simulink model "CDF_VSVPWM_Grid_Parallel"  (R2024b) (continued)
== system CDF_VSVPWM_Grid_Parallel/VSVPWM == (continued)
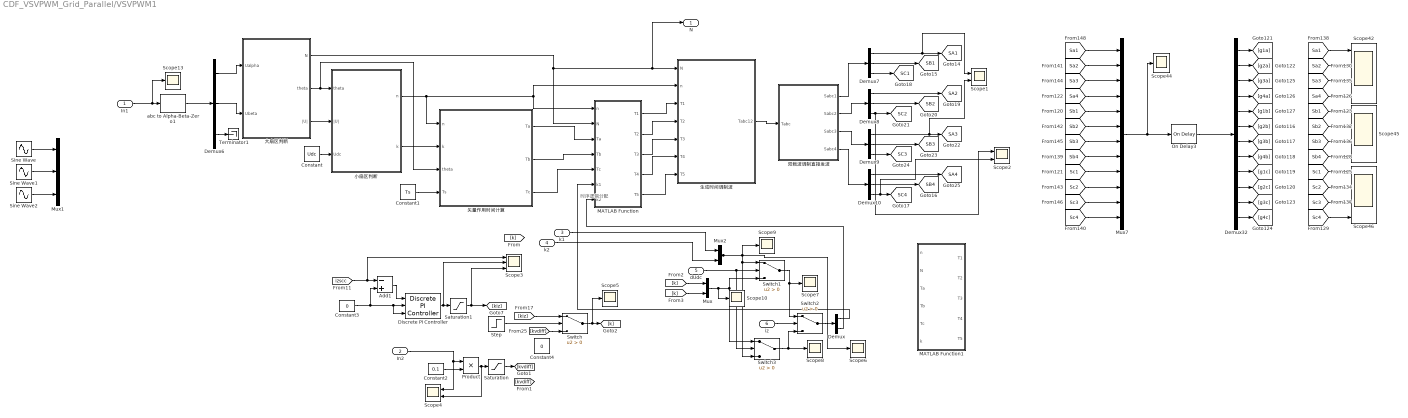
== system CDF_VSVPWM_Grid_Parallel/VSVPWM1 ==
  note: 时序逻辑分配
  In1 : Inport
  In2 : Inport  Port=2
  k1 : Inport  Port=3
  k2 : Inport  Port=4
  dUdc : Inport  Port=5
  iz : Inport  Port=6
  Add1 : Sum  Inputs=-+
  Constant : Constant  Value=Udc
  Constant1 : Constant  Value=Ts
  Constant2 : Constant  Value=0.1
  Constant3 : Constant  Value=0
  Constant4 : Constant  Value=0
  Demux : Demux  Outputs=2
  Demux{6..10} (5 alike) : Demux  Outputs=3
  Demux32 : Demux  Outputs=12
  Discrete PI Controller : eeDiscretePi/Discrete PI Controller  Kp=5  Ki=0  Kaw=1  x0=0  Ts=-1
  MATLAB Function : MATLAB Function  TreatAsAtomicUnit=on
  MATLAB Function1 : MATLAB Function  TreatAsAtomicUnit=on
  Mux : Mux  Inputs=2
  Mux1 : Mux  Inputs=3
  Mux2 : Mux  Inputs=2
  Mux7 : Mux  Inputs=12
  On Delay3 : spsOnDelayLib/On Delay  DelayType=On delay  delay=250e-9  ic=0  Ts=0
  Product : Product
  Saturation : Saturate  UpperLimit=1  LowerLimit=-1
  Saturation1 : Saturate  UpperLimit=1  LowerLimit=-1
  Scope1 : Scope  NumInputPorts=2
  Scope{5..10,13,44} (8 alike) : Scope
  Scope2 : Scope  NumInputPorts=2
  Scope3 : Scope  NumInputPorts=3
  Scope4 : Scope  NumInputPorts=2
  Scope42 : Scope  NumInputPorts=4
  Scope45 : Scope  NumInputPorts=4
  Scope46 : Scope  NumInputPorts=4
  Sine Wave : Sin  Amplitude=m*Udc/sqrt(3)  Frequency=100*pi  SampleTime=0
  Sine Wave1 : Sin  Amplitude=m*Udc/sqrt(3)  Frequency=100*pi  Phase=-2*pi/3  SampleTime=0
  Sine Wave2 : Sin  Amplitude=m*Udc/sqrt(3)  Frequency=100*pi  Phase=2*pi/3  SampleTime=0
  Step : Step  SampleTime=0
  Switch{,1..3} (4 alike) : Switch  Criteria=u2 > Threshold  SaturateOnIntegerOverflow=off  InputSameDT=off
  Terminator1 : Terminator
  abc to Alpha-Beta-Zero1 : spsabctoAlphaBetaZeroLib/abc to Alpha-Beta-Zero
  双载波调制直接发波 : Subsystem
  大扇区判断 : Subsystem
  小扇区判断 : Subsystem
  生成时间调制波 : Subsystem
  矢量作用时间计算 : Subsystem
  N : Outport
  tag scope: SA1=global, SA2=global, SA3=global, SA4=global, SB1=global, SB2=global, SB3=global, SB4=global, SC1=global, SC2=global, SC3=global, SC4=global
  wires:
  大扇区判断/2(theta) -> 矢量作用时间计算/3(theta), 小扇区判断/1(theta)
  大扇区判断/3(|U|) -> 小扇区判断/2(|U|)
  Constant/1 -> 小扇区判断/3(Udc)
  Demux6/2 -> 大扇区判断/2(Ubeta)
  abc to Alpha-Beta-Zero1/1 -> Demux6/1
  In1/1 -> Scope13/1, abc to Alpha-Beta-Zero1/1
  Demux6/1 -> 大扇区判断/1(Ualpha)
  小扇区判断/2(k) -> 矢量作用时间计算/2(k)
  小扇区判断/1(n) -> 矢量作用时间计算/1(n), MATLAB Function/1(n), 生成时间调制波/2(n)
  Constant1/1 -> 矢量作用时间计算/4(Ts)
  大扇区判断/1(N) -> MATLAB Function/2(N), N/1, 生成时间调制波/1(N)
  生成时间调制波/1(Tabc12) -> 双载波调制直接发波/1(Tabc)
  Demux9/3 -> [SC3]
  Demux9/2 -> [SB3]
  Demux8/1 -> [SA2]
  Demux10/2 -> [SB4]
  Demux10/3 -> Scope2/2, [SC4]
  Demux7/1 -> Scope1/1, [SA1]
  Demux10/1 -> [SA4]
  Demux8/2 -> [SB2]
  Demux7/2 -> [SB1]
  Demux7/3 -> [SC1]
  Demux9/1 -> Scope1/2, [SA3]
  Demux8/3 -> Scope2/1, [SC2]
  双载波调制直接发波/1(Sabc1) -> Demux7/1
  双载波调制直接发波/2(Sabc2) -> Demux8/1
  双载波调制直接发波/3(Sabc3) -> Demux9/1
  双载波调制直接发波/4(Sabc4) -> Demux10/1
  Demux6/3 -> Terminator1/1
  [Sc3] -> Scope46/3
  [Sa3] -> Scope42/3
  Demux32/11 -> [g3c]
  Demux32/2 -> [g2a]
  On Delay3/1 -> Demux32/1
  Demux32/5 -> [g1b]
  Demux32/12 -> [g4c]
  Demux32/1 -> [g1a]
  Demux32/10 -> [g2c]
  Demux32/7 -> [g3b]
  Demux32/8 -> [g4b]
  Demux32/6 -> [g2b]
  [Sb3] -> Mux7/7
  Mux7/1 -> On Delay3/1, Scope44/1
  [Sc1] -> Mux7/9
  [Sb1] -> Scope45/1
  [Sb2] -> Scope45/2
  [Sa2] -> Scope42/2
  [Sb3] -> Scope45/3
  Demux32/9 -> [g1c]
  [Sb4] -> Scope45/4
  Demux32/3 -> [g3a]
  [Sb1] -> Mux7/5
  [Sa1] -> Mux7/1
  [Sa3] -> Mux7/3
  [Sb2] -> Mux7/6
  [Sa2] -> Mux7/2
  [Sb4] -> Mux7/8
  Demux32/4 -> [g4a]
  [Sa4] -> Mux7/4
  [Sc4] -> Mux7/12
  [Sc4] -> Scope46/4
  [Sc2] -> Scope46/2
  [Sa1] -> Scope42/1
  [Sc3] -> Mux7/11
  [Sc2] -> Mux7/10
  [Sa4] -> Scope42/4
  [Sc1] -> Scope46/1
  Sine Wave1/1 -> Mux1/2
  Sine Wave2/1 -> Mux1/3
  Sine Wave/1 -> Mux1/1
  Saturation/1 -> [kvdiff]
  Add1/1 -> Discrete PI Controller/1
  Switch/1 -> Scope5/1, [k]
  Constant2/1 -> Product/2
  In2/1 -> Scope4/1, Product/1
  Step/1 -> Switch/2
  Discrete PI Controller/1 -> Scope3/2, Saturation1/1
  Saturation1/1 -> Scope3/3, [kiz]
  Product/1 -> Scope4/2, Saturation/1
  Constant3/1 -> Add1/2, Discrete PI Controller/3, Discrete PI Controller/2
  [izscc] -> Scope3/1, Add1/1
  [kiz] -> Switch/1
  [kvdiff] -> Switch/3
  矢量作用时间计算/1(Ta) -> MATLAB Function/3(Ta)
  矢量作用时间计算/2(Tb) -> MATLAB Function/4(Tb)
  矢量作用时间计算/3(Tc) -> MATLAB Function/5(Tc)
  MATLAB Function/1(T1) -> 生成时间调制波/3(T1)
  MATLAB Function/2(T2) -> 生成时间调制波/4(T2)
  MATLAB Function/3(T3) -> 生成时间调制波/5(T3)
  MATLAB Function/4(T4) -> 生成时间调制波/6(T4)
  MATLAB Function/5(T5) -> 生成时间调制波/7(T5)
  iz/1 -> Switch2/2
  [k] -> Mux/1
  Switch1/1 -> Switch2/1, Scope7/1
  Mux/1 -> Scope10/1, Switch3/1, Switch1/3
  Mux2/1 -> Switch3/3, Switch1/1, Scope9/1, Demux/1
  Switch3/1 -> Scope8/1, Switch2/3
  [k] -> Mux/2
  Switch2/1 -> Scope6/1
  dUdc/1 -> Switch1/2, Switch3/2
  Demux/1 -> MATLAB Function/6(k1)
  Demux/2 -> MATLAB Function/7(k2)
  k1/1 -> Mux2/1
  k2/1 -> Mux2/2

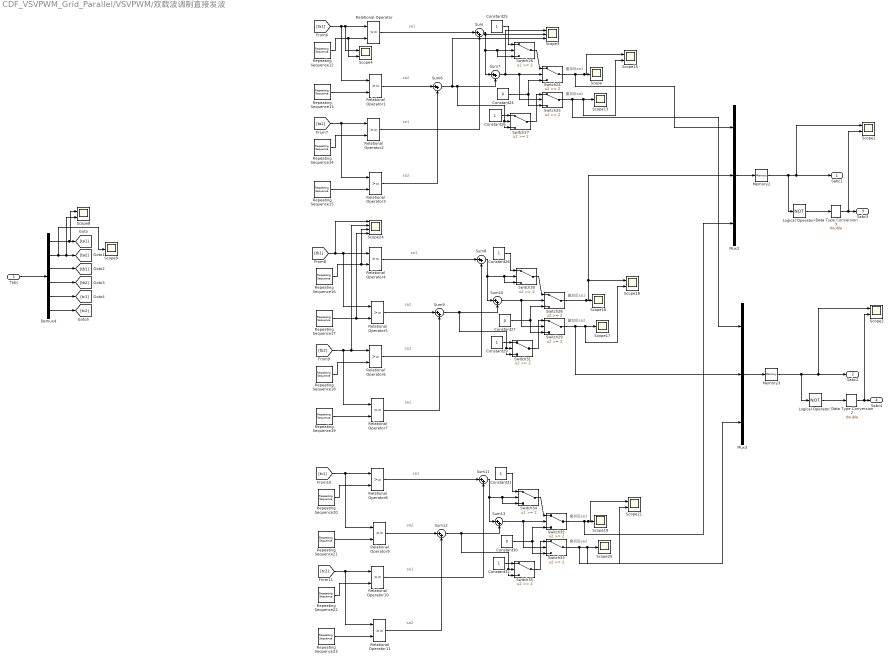
== system CDF_VSVPWM_Grid_Parallel/VSVPWM/双载波调制直接发波 ==
  note: 叠加后sa1
  note: sa1
  note: sa2
  note: sa1
  note: sa2
  note: 叠加后sa2
  note: 叠加后sa1
  note: 叠加后sa2
  note: sa1
  note: sa1
  note: sa2
  note: sa2
  note: 叠加后sa1
  note: 叠加后sa2
  note: sa1
  note: sa1
  note: sa2
  note: sa2
  Tabc : Inport
  Constant24 : Constant  Value=0
  Constant{25..26,28..29,31..32} (6 alike) : Constant
  Constant27 : Constant  Value=0
  Constant30 : Constant  Value=0
  Data Type Conversion2 : DataTypeConversion  OutDataTypeStr=double  SaturateOnIntegerOverflow=off  RndMeth=Floor
  Data Type Conversion3 : DataTypeConversion  OutDataTypeStr=double  SaturateOnIntegerOverflow=off  RndMeth=Floor
  Demux4 : Demux  Outputs=6
  Logical Operator1 : Logic  Operator=NOT  AllPortsSameDT=off  OutDataTypeStr=boolean
  Logical Operator4 : Logic  Operator=NOT  AllPortsSameDT=off  OutDataTypeStr=boolean
  Memory2 : Memory
  Memory3 : Memory
  Mux2 : Mux  Inputs=3
  Mux3 : Mux  Inputs=3
  Relational Operator{,1..11} (12 alike) : RelationalOperator  OutDataTypeStr=boolean  RndMeth=Simplest  InputSameDT=off
  Repeating Sequence{12,14,16,18,20,22} (6 alike) : simulink/Sources/Repeating Sequence  rep_seq_t=[0 Ts/2 Ts]  rep_seq_y=[0 1 0]
  Repeating Sequence{13,15,17,19,21,23} (6 alike) : simulink/Sources/Repeating Sequence  rep_seq_t=[0 Ts/2 Ts]  rep_seq_y=[-1 0 -1]
  Scope{,9,13,16..17,19..20} (7 alike) : Scope
  Scope{1..2,4,8,15,18,21} (7 alike) : Scope  NumInputPorts=2
  Scope24 : Scope  NumInputPorts=4
  Scope3 : Scope  NumInputPorts=3
  Sum{,6..13} (9 alike) : Sum  Inputs=|++
  Switch{24..35} (12 alike) : Switch  Threshold=2  SaturateOnIntegerOverflow=off  InputSameDT=off
  Sabc1 : Outport
  Sabc2 : Outport  Port=2
  Sabc3 : Outport  Port=3
  Sabc4 : Outport  Port=4
  wires:
  Tabc/1 -> Demux4/1
  Demux4/1 -> Scope8/1, [ta1]
  Demux4/2 -> Scope9/1, Scope8/2, [ta2]
  Demux4/3 -> [tb1]
  Demux4/4 -> [tb2]
  Demux4/5 -> [tc1]
  Demux4/6 -> [tc2]
  [ta1] -> Relational Operator1/1, Scope4/1, Relational Operator/1
  Repeating Sequence12/1 -> Scope4/2, Relational Operator/2
  Repeating Sequence13/1 -> Relational Operator1/2
  Relational Operator/1 -> Sum/1
  Relational Operator1/1 -> Sum6/1
  Relational Operator2/1 -> Sum/2
  [ta2] -> Relational Operator3/1, Relational Operator2/1
  Repeating Sequence15/1 -> Relational Operator3/2
  Repeating Sequence14/1 -> Relational Operator2/2
  Relational Operator3/1 -> Sum6/2
  Sum/1 -> Switch26/3, Switch26/2, Sum7/1, Scope3/2
  Sum6/1 -> Scope3/3, Switch27/3, Switch27/2, Sum7/2
  Sum7/1 -> Scope3/1, Switch25/2, Switch24/2
  Constant24/1 -> Switch25/3, Switch24/3
  Switch24/1 -> Mux2/1, Scope15/1, Scope/1
  Switch25/1 -> Mux3/1, Scope15/2, Scope13/1
  Constant25/1 -> Switch26/1
  Switch26/1 -> Switch24/1
  Switch27/1 -> Switch25/1
  Constant26/1 -> Switch27/1
  Switch28/1 -> Mux2/2, Scope18/1, Scope16/1
  Switch30/1 -> Switch28/1
  Constant28/1 -> Switch30/1
  Relational Operator7/1 -> Sum9/2
  Sum8/1 -> Switch30/3, Switch30/2, Sum10/1
  Relational Operator4/1 -> Sum8/1
  Repeating Sequence17/1 -> Relational Operator5/2, Scope24/4
  Sum10/1 -> Switch29/2, Switch28/2
  [tb1] -> Scope24/1, Relational Operator5/1, Relational Operator4/1
  Relational Operator5/1 -> Sum9/1
  Switch29/1 -> Mux3/2, Scope18/2, Scope17/1
  Repeating Sequence16/1 -> Scope24/3, Relational Operator4/2
  Sum9/1 -> Switch31/3, Switch31/2, Sum10/2
  Relational Operator6/1 -> Sum8/2
  Repeating Sequence18/1 -> Relational Operator6/2
  Constant27/1 -> Switch29/3, Switch28/3
  Repeating Sequence19/1 -> Relational Operator7/2
  [tb2] -> Scope24/2, Relational Operator6/1, Relational Operator7/1
  Switch31/1 -> Switch29/1
  Constant29/1 -> Switch31/1
  Switch32/1 -> Mux2/3, Scope21/1, Scope19/1
  Switch34/1 -> Switch32/1
  Constant31/1 -> Switch34/1
  Relational Operator11/1 -> Sum12/2
  Sum11/1 -> Switch34/3, Switch34/2, Sum13/1
  Relational Operator8/1 -> Sum11/1
  Repeating Sequence21/1 -> Relational Operator9/2
  Sum13/1 -> Switch33/2, Switch32/2
  [tc1] -> Relational Operator9/1, Relational Operator8/1
  Relational Operator9/1 -> Sum12/1
  Switch33/1 -> Mux3/3, Scope21/2, Scope20/1
  Repeating Sequence20/1 -> Relational Operator8/2
  Sum12/1 -> Switch35/3, Switch35/2, Sum13/2
  Relational Operator10/1 -> Sum11/2
  Repeating Sequence22/1 -> Relational Operator10/2
  Constant30/1 -> Switch33/3, Switch32/3
  Repeating Sequence23/1 -> Relational Operator11/2
  [tc2] -> Relational Operator11/1, Relational Operator10/1
  Switch35/1 -> Switch33/1
  Constant32/1 -> Switch35/1
  Logical Operator1/1 -> Data Type Conversion2/1
  Logical Operator4/1 -> Data Type Conversion3/1
  Mux2/1 -> Memory2/1
  Data Type Conversion3/1 -> Scope1/2, Sabc3/1
  Mux3/1 -> Memory3/1
  Data Type Conversion2/1 -> Scope2/2, Sabc4/1
  Memory2/1 -> Logical Operator4/1, Scope1/1, Sabc1/1
  Memory3/1 -> Logical Operator1/1, Scope2/1, Sabc2/1

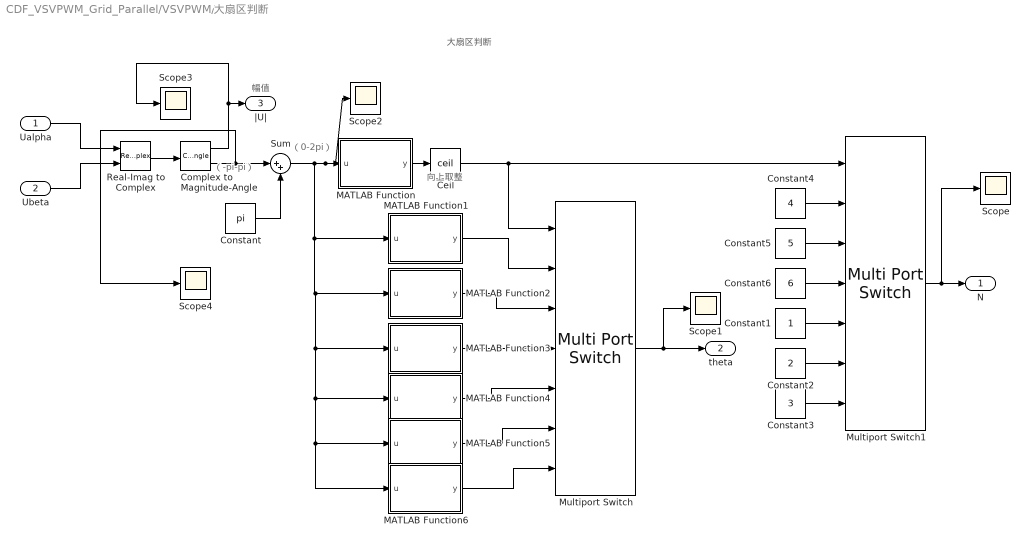
== system CDF_VSVPWM_Grid_Parallel/VSVPWM/大扇区判断 ==
  note: 幅值
  note: 向上取整
  note: 大扇区判断
  note: （0-2pi）
  note: （-pi-pi）
  Ualpha : Inport
  Ubeta : Inport  Port=2
  Ceil : Rounding  Operator=ceil
  Complex to Magnitude-Angle : ComplexToMagnitudeAngle
  Constant : Constant  Value=pi
  Constant1 : Constant
  Constant2 : Constant  Value=2
  Constant3 : Constant  Value=3
  Constant4 : Constant  Value=4
  Constant5 : Constant  Value=5
  Constant6 : Constant  Value=6
  MATLAB Function : MATLAB Function  TreatAsAtomicUnit=on
  MATLAB Function1 : MATLAB Function  TreatAsAtomicUnit=on
  MATLAB Function2 : MATLAB Function  TreatAsAtomicUnit=on
  MATLAB Function3 : MATLAB Function  TreatAsAtomicUnit=on
  MATLAB Function4 : MATLAB Function  TreatAsAtomicUnit=on
  MATLAB Function5 : MATLAB Function  TreatAsAtomicUnit=on
  MATLAB Function6 : MATLAB Function  TreatAsAtomicUnit=on
  Multiport Switch : MultiPortSwitch  Inputs=6  SaturateOnIntegerOverflow=off  InputSameDT=off
  Multiport Switch1 : MultiPortSwitch  Inputs=6  SaturateOnIntegerOverflow=off  InputSameDT=off
  Real-Imag to Complex : RealImagToComplex
  Scope{,1..4} (5 alike) : Scope
  Sum : Sum  Inputs=|++
  N : Outport
  theta : Outport  Port=2
  |U| : Outport  Port=3
  wires:
  Ualpha/1 -> Real-Imag to Complex/1
  Ubeta/1 -> Real-Imag to Complex/2
  Real-Imag to Complex/1 -> Complex to Magnitude-Angle/1
  Complex to Magnitude-Angle/2 -> Scope4/1, Sum/1
  Constant/1 -> Sum/2
  Sum/1 -> Scope2/1, MATLAB Function/1(u), MATLAB Function6/1(u), MATLAB Function5/1(u), MATLAB Function4/1(u), MATLAB Function3/1(u), MATLAB Function2/1(u), MATLAB Function1/1(u)
  MATLAB Function/1(y) -> Ceil/1
  Ceil/1 -> Multiport Switch1/1, Multiport Switch/1
  Multiport Switch/1 -> Scope1/1, theta/1
  MATLAB Function1/1(y) -> Multiport Switch/2
  MATLAB Function2/1(y) -> Multiport Switch/3
  MATLAB Function3/1(y) -> Multiport Switch/4
  MATLAB Function4/1(y) -> Multiport Switch/5
  MATLAB Function5/1(y) -> Multiport Switch/6
  MATLAB Function6/1(y) -> Multiport Switch/7
  Multiport Switch1/1 -> Scope/1, N/1
  Constant1/1 -> Multiport Switch1/5
  Constant2/1 -> Multiport Switch1/6
  Constant3/1 -> Multiport Switch1/7
  Constant4/1 -> Multiport Switch1/2
  Constant5/1 -> Multiport Switch1/3
  Constant6/1 -> Multiport Switch1/4
  Complex to Magnitude-Angle/1 -> |U|/1, Scope3/1

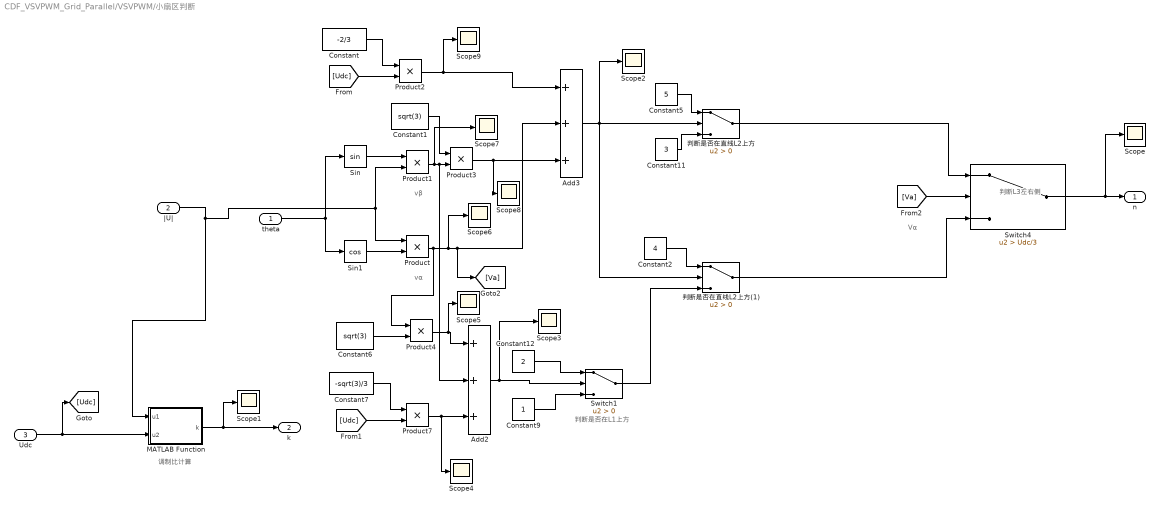
== system CDF_VSVPWM_Grid_Parallel/VSVPWM/小扇区判断 ==
  note: Vα
  note: 判断L3左右侧
  note: vα
  note: 调制比计算
  note: vβ
  note: 判断是否在L1上方
  theta : Inport
  |U| : Inport  Port=2
  Udc : Inport  Port=3
  "   判断是否在直线L2上方" : Switch  Criteria=u2 > Threshold  SaturateOnIntegerOverflow=off  InputSameDT=off
  "   判断是否在直线L2上方(1)" : Switch  Criteria=u2 > Threshold  SaturateOnIntegerOverflow=off  InputSameDT=off
  Add2 : Sum  Inputs=+++
  Add3 : Sum  Inputs=+++
  Constant : Constant  Value=-2/3
  Constant1 : Constant  Value=sqrt(3)
  Constant11 : Constant  Value=3
  Constant12 : Constant  Value=2
  Constant2 : Constant  Value=4
  Constant5 : Constant  Value=5
  Constant6 : Constant  Value=sqrt(3)
  Constant7 : Constant  Value=-sqrt(3)/3
  Constant9 : Constant
  MATLAB Function : MATLAB Function  TreatAsAtomicUnit=on
  Product{,1..4,7} (6 alike) : Product
  Scope{,1..9} (10 alike) : Scope
  Sin : Trigonometry
  Sin1 : Trigonometry  Operator=cos
  Switch1 : Switch  Criteria=u2 > Threshold  SaturateOnIntegerOverflow=off  InputSameDT=off
  Switch4 : Switch  Criteria=u2 > Threshold  Threshold=Udc/3  SaturateOnIntegerOverflow=off  InputSameDT=off
  n : Outport
  k : Outport  Port=2
  wires:
  Udc/1 -> [Udc], MATLAB Function/2(u2)
  |U|/1 -> MATLAB Function/1(u1), Product1/2, Product/1
  MATLAB Function/1(k) -> Scope1/1, k/1
  theta/1 -> Sin1/1, Sin/1
  Constant/1 -> Product2/1
  [Udc] -> Product2/2
  Constant5/1 -> "   判断是否在直线L2上方"/1
  Switch4/1 -> Scope/1, n/1
  Sin/1 -> Product1/1
  Sin1/1 -> Product/2
  Constant11/1 -> "   判断是否在直线L2上方"/3
  Product1/1 -> Add2/2, Product3/2, Scope7/1
  Constant1/1 -> Product3/1
  Product3/1 -> Scope8/1, Add3/3
  Product/1 -> Scope6/1, [Va], Add3/2, Product4/1
  Product2/1 -> Scope9/1, Add3/1
  Add3/1 -> "   判断是否在直线L2上方(1)"/2, Scope2/1, "   判断是否在直线L2上方"/2
  "   判断是否在直线L2上方"/1 -> Switch4/1
  Constant2/1 -> "   判断是否在直线L2上方(1)"/1
  Constant12/1 -> Switch1/1
  Constant9/1 -> Switch1/3
  Constant6/1 -> Product4/2
  Product4/1 -> Scope5/1, Add2/1
  [Udc] -> Product7/2
  Constant7/1 -> Product7/1
  Product7/1 -> Scope4/1, Add2/3
  Add2/1 -> Scope3/1, Switch1/2
  Switch1/1 -> "   判断是否在直线L2上方(1)"/3
  "   判断是否在直线L2上方(1)"/1 -> Switch4/3
  [Va] -> Switch4/2

== system CDF_VSVPWM_Grid_Parallel/VSVPWM/生成时间调制波 ==
  note: 调制信号生成
  note: 小扇区
  note: 大扇区
  N : Inport
  n : Inport  Port=2
  T1 : Inport  Port=3
  T2 : Inport  Port=4
  T3 : Inport  Port=5
  T4 : Inport  Port=6
  T5 : Inport  Port=7
  Demux : Demux  Outputs=6
  Gain : Gain  Gain=1/Ts
  Multiport Switch : MultiPortSwitch  Inputs=6  SaturateOnIntegerOverflow=off  InputSameDT=off
  Mux : Mux  Inputs=5
  Scope10 : Scope  NumInputPorts=6
  Scope11 : Scope  NumInputPorts=3
  Scope12 : Scope  NumInputPorts=3
  Scope13 : Scope  NumInputPorts=3
  Scope6 : Scope
  Scope9 : Scope  NumInputPorts=2
  Ⅰ扇区的调制信号计算 : Subsystem
  Ⅱ扇区的调制信号计算 : Subsystem
  Ⅲ扇区的调制信号计算1 : Subsystem
  Ⅳ扇区的调制信号计算 : Subsystem
  Ⅴ扇区的调制信号计算 : Subsystem
  Ⅵ扇区的调制信号计算 : Subsystem
  Tabc12 : Outport
  wires:
  T2/1 -> Mux/2
  T3/1 -> Mux/3
  T1/1 -> Mux/1
  Mux/1 -> Ⅳ扇区的调制信号计算/2(T12345), Ⅵ扇区的调制信号计算/2(T12345), Ⅴ扇区的调制信号计算/2(T12345), Ⅲ扇区的调制信号计算1/2(T12345), Ⅱ扇区的调制信号计算/2(T12345), Ⅰ扇区的调制信号计算/2(T12345)
  n/1 -> Ⅵ扇区的调制信号计算/1(n), Ⅴ扇区的调制信号计算/1(n), Ⅳ扇区的调制信号计算/1(n), Ⅲ扇区的调制信号计算1/1(n), Ⅱ扇区的调制信号计算/1(n), Ⅰ扇区的调制信号计算/1(n)
  Ⅰ扇区的调制信号计算/1(Tabc12) -> Scope12/2, Multiport Switch/2
  Ⅱ扇区的调制信号计算/1(Tabc12) -> Scope11/2, Multiport Switch/3
  N/1 -> Scope13/2, Scope11/1, Scope12/1, Scope9/2, Multiport Switch/1
  Ⅲ扇区的调制信号计算1/1(Tabc12) -> Scope13/1, Multiport Switch/4
  Ⅴ扇区的调制信号计算/1(Tabc12) -> Multiport Switch/6
  Ⅳ扇区的调制信号计算/1(Tabc12) -> Multiport Switch/5
  Ⅵ扇区的调制信号计算/1(Tabc12) -> Scope6/1, Multiport Switch/7
  T4/1 -> Mux/4
  T5/1 -> Mux/5
  ? -> ?
  Gain/1 -> Scope9/1, Tabc12/1
  Multiport Switch/1 -> Scope13/3, Scope11/3, Scope12/3, Demux/1, Gain/1
  Demux/1 -> Scope10/1
  Demux/2 -> Scope10/2
  Demux/3 -> Scope10/3
  Demux/4 -> Scope10/4
  Demux/5 -> Scope10/5
  Demux/6 -> Scope10/6

== system CDF_VSVPWM_Grid_Parallel/VSVPWM/矢量作用时间计算 ==
  note: 矢量作用时长
  note: 小扇区
  note: 目标矢量角度
  note: 采样时间
  note: 调制比
  n : Inport
  k : Inport  Port=2
  theta : Inport  Port=3
  Ts : Inport  Port=4
  Demux : Demux  Outputs=3
  I1 : Subsystem
  I2 : Subsystem
  I3 : Subsystem
  I4 : Subsystem
  I5 : Subsystem
  Multiport Switch : MultiPortSwitch  Inputs=5  SaturateOnIntegerOverflow=off  InputSameDT=off
  Scope : Scope  NumInputPorts=3
  Ta : Outport
  Tb : Outport  Port=2
  Tc : Outport  Port=3
  wires:
  theta/1 -> I2/3(theta), I3/3(theta), I5/3(theta), I4/3(theta), I1/3(theta)
  Ts/1 -> I2/2(Ts), I3/2(Ts), I5/2(Ts), I4/2(Ts), I1/2(Ts)
  k/1 -> I2/1(k), I3/1(k), I5/1(k), I4/1(k), I1/1(k)
  n/1 -> Multiport Switch/1
  Multiport Switch/1 -> Demux/1
  I1/1(T) -> Multiport Switch/2
  I2/1(T) -> Multiport Switch/3
  I3/1(T) -> Multiport Switch/4
  I4/1(T) -> Multiport Switch/5
  I5/1(T) -> Multiport Switch/6
  Demux/1 -> Scope/1, Ta/1
  Demux/2 -> Scope/2, Tb/1
  Demux/3 -> Scope/3, Tc/1

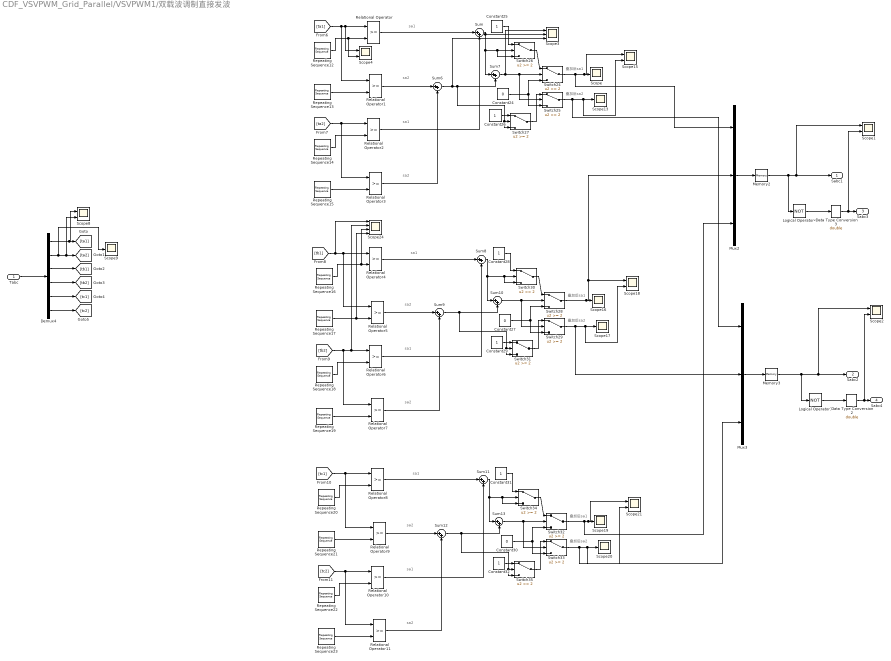
== system CDF_VSVPWM_Grid_Parallel/VSVPWM1/双载波调制直接发波 ==
  note: 叠加后sa1
  note: sa1
  note: sa2
  note: sa1
  note: sa2
  note: 叠加后sa2
  note: 叠加后sa1
  note: 叠加后sa2
  note: sa1
  note: sa1
  note: sa2
  note: sa2
  note: 叠加后sa1
  note: 叠加后sa2
  note: sa1
  note: sa1
  note: sa2
  note: sa2
  Tabc : Inport
  Constant24 : Constant  Value=0
  Constant{25..26,28..29,31..32} (6 alike) : Constant
  Constant27 : Constant  Value=0
  Constant30 : Constant  Value=0
  Data Type Conversion2 : DataTypeConversion  OutDataTypeStr=double  SaturateOnIntegerOverflow=off  RndMeth=Floor
  Data Type Conversion3 : DataTypeConversion  OutDataTypeStr=double  SaturateOnIntegerOverflow=off  RndMeth=Floor
  Demux4 : Demux  Outputs=6
  Logical Operator1 : Logic  Operator=NOT  AllPortsSameDT=off  OutDataTypeStr=boolean
  Logical Operator4 : Logic  Operator=NOT  AllPortsSameDT=off  OutDataTypeStr=boolean
  Memory2 : Memory
  Memory3 : Memory
  Mux2 : Mux  Inputs=3
  Mux3 : Mux  Inputs=3
  Relational Operator{,1..11} (12 alike) : RelationalOperator  OutDataTypeStr=boolean  RndMeth=Simplest  InputSameDT=off
  Repeating Sequence{12,14,16,18,20,22} (6 alike) : simulink/Sources/Repeating Sequence  rep_seq_t=[0 Ts/2 Ts]  rep_seq_y=[0 1 0]
  Repeating Sequence{13,15,17,19,21,23} (6 alike) : simulink/Sources/Repeating Sequence  rep_seq_t=[0 Ts/2 Ts]  rep_seq_y=[-1 0 -1]
  Scope{,9,13,16..17,19..20} (7 alike) : Scope
  Scope{1..2,4,8,15,18,21} (7 alike) : Scope  NumInputPorts=2
  Scope24 : Scope  NumInputPorts=4
  Scope3 : Scope  NumInputPorts=3
  Sum{,6..13} (9 alike) : Sum  Inputs=|++
  Switch{24..35} (12 alike) : Switch  Threshold=2  SaturateOnIntegerOverflow=off  InputSameDT=off
  Sabc1 : Outport
  Sabc2 : Outport  Port=2
  Sabc3 : Outport  Port=3
  Sabc4 : Outport  Port=4
  wires:
  Tabc/1 -> Demux4/1
  Demux4/1 -> Scope8/1, [ta1]
  Demux4/2 -> Scope9/1, Scope8/2, [ta2]
  Demux4/3 -> [tb1]
  Demux4/4 -> [tb2]
  Demux4/5 -> [tc1]
  Demux4/6 -> [tc2]
  [ta1] -> Relational Operator1/1, Scope4/1, Relational Operator/1
  Repeating Sequence12/1 -> Scope4/2, Relational Operator/2
  Repeating Sequence13/1 -> Relational Operator1/2
  Relational Operator/1 -> Sum/1
  Relational Operator1/1 -> Sum6/1
  Relational Operator2/1 -> Sum/2
  [ta2] -> Relational Operator3/1, Relational Operator2/1
  Repeating Sequence15/1 -> Relational Operator3/2
  Repeating Sequence14/1 -> Relational Operator2/2
  Relational Operator3/1 -> Sum6/2
  Sum/1 -> Switch26/3, Switch26/2, Sum7/1, Scope3/2
  Sum6/1 -> Scope3/3, Switch27/3, Switch27/2, Sum7/2
  Sum7/1 -> Scope3/1, Switch25/2, Switch24/2
  Constant24/1 -> Switch25/3, Switch24/3
  Switch24/1 -> Mux2/1, Scope15/1, Scope/1
  Switch25/1 -> Mux3/1, Scope15/2, Scope13/1
  Constant25/1 -> Switch26/1
  Switch26/1 -> Switch24/1
  Switch27/1 -> Switch25/1
  Constant26/1 -> Switch27/1
  Switch28/1 -> Mux2/2, Scope18/1, Scope16/1
  Switch30/1 -> Switch28/1
  Constant28/1 -> Switch30/1
  Relational Operator7/1 -> Sum9/2
  Sum8/1 -> Switch30/3, Switch30/2, Sum10/1
  Relational Operator4/1 -> Sum8/1
  Repeating Sequence17/1 -> Relational Operator5/2, Scope24/4
  Sum10/1 -> Switch29/2, Switch28/2
  [tb1] -> Scope24/1, Relational Operator5/1, Relational Operator4/1
  Relational Operator5/1 -> Sum9/1
  Switch29/1 -> Mux3/2, Scope18/2, Scope17/1
  Repeating Sequence16/1 -> Scope24/3, Relational Operator4/2
  Sum9/1 -> Switch31/3, Switch31/2, Sum10/2
  Relational Operator6/1 -> Sum8/2
  Repeating Sequence18/1 -> Relational Operator6/2
  Constant27/1 -> Switch29/3, Switch28/3
  Repeating Sequence19/1 -> Relational Operator7/2
  [tb2] -> Scope24/2, Relational Operator6/1, Relational Operator7/1
  Switch31/1 -> Switch29/1
  Constant29/1 -> Switch31/1
  Switch32/1 -> Mux2/3, Scope21/1, Scope19/1
  Switch34/1 -> Switch32/1
  Constant31/1 -> Switch34/1
  Relational Operator11/1 -> Sum12/2
  Sum11/1 -> Switch34/3, Switch34/2, Sum13/1
  Relational Operator8/1 -> Sum11/1
  Repeating Sequence21/1 -> Relational Operator9/2
  Sum13/1 -> Switch33/2, Switch32/2
  [tc1] -> Relational Operator9/1, Relational Operator8/1
  Relational Operator9/1 -> Sum12/1
  Switch33/1 -> Mux3/3, Scope21/2, Scope20/1
  Repeating Sequence20/1 -> Relational Operator8/2
  Sum12/1 -> Switch35/3, Switch35/2, Sum13/2
  Relational Operator10/1 -> Sum11/2
  Repeating Sequence22/1 -> Relational Operator10/2
  Constant30/1 -> Switch33/3, Switch32/3
  Repeating Sequence23/1 -> Relational Operator11/2
  [tc2] -> Relational Operator11/1, Relational Operator10/1
  Switch35/1 -> Switch33/1
  Constant32/1 -> Switch35/1
  Logical Operator1/1 -> Data Type Conversion2/1
  Logical Operator4/1 -> Data Type Conversion3/1
  Mux2/1 -> Memory2/1
  Data Type Conversion3/1 -> Scope1/2, Sabc3/1
  Mux3/1 -> Memory3/1
  Data Type Conversion2/1 -> Scope2/2, Sabc4/1
  Memory2/1 -> Logical Operator4/1, Scope1/1, Sabc1/1
  Memory3/1 -> Logical Operator1/1, Scope2/1, Sabc2/1

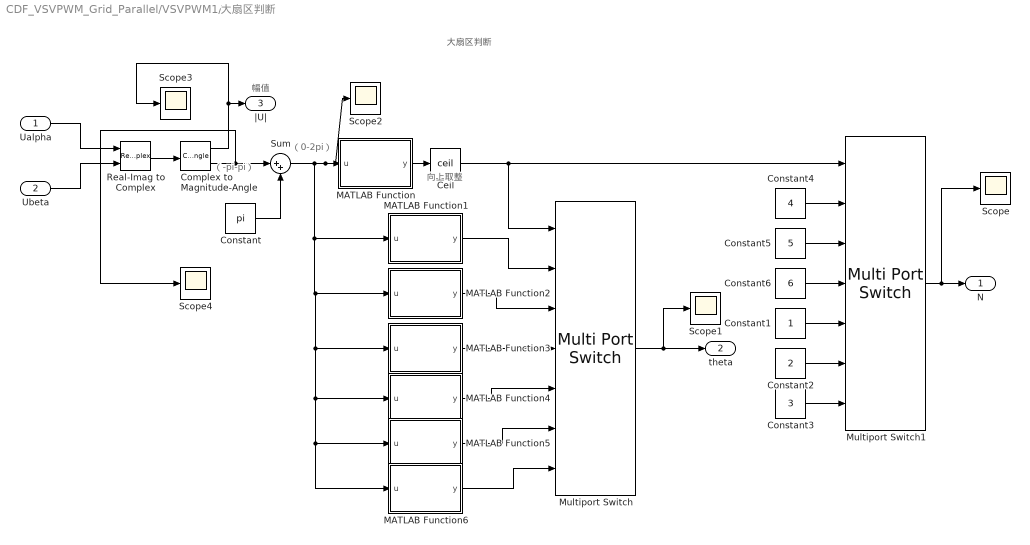
== system CDF_VSVPWM_Grid_Parallel/VSVPWM1/大扇区判断 ==
  note: 幅值
  note: 向上取整
  note: 大扇区判断
  note: （0-2pi）
  note: （-pi-pi）
  Ualpha : Inport
  Ubeta : Inport  Port=2
  Ceil : Rounding  Operator=ceil
  Complex to Magnitude-Angle : ComplexToMagnitudeAngle
  Constant : Constant  Value=pi
  Constant1 : Constant
  Constant2 : Constant  Value=2
  Constant3 : Constant  Value=3
  Constant4 : Constant  Value=4
  Constant5 : Constant  Value=5
  Constant6 : Constant  Value=6
  MATLAB Function : MATLAB Function  TreatAsAtomicUnit=on
  MATLAB Function1 : MATLAB Function  TreatAsAtomicUnit=on
  MATLAB Function2 : MATLAB Function  TreatAsAtomicUnit=on
  MATLAB Function3 : MATLAB Function  TreatAsAtomicUnit=on
  MATLAB Function4 : MATLAB Function  TreatAsAtomicUnit=on
  MATLAB Function5 : MATLAB Function  TreatAsAtomicUnit=on
  MATLAB Function6 : MATLAB Function  TreatAsAtomicUnit=on
  Multiport Switch : MultiPortSwitch  Inputs=6  SaturateOnIntegerOverflow=off  InputSameDT=off
  Multiport Switch1 : MultiPortSwitch  Inputs=6  SaturateOnIntegerOverflow=off  InputSameDT=off
  Real-Imag to Complex : RealImagToComplex
  Scope{,1..4} (5 alike) : Scope
  Sum : Sum  Inputs=|++
  N : Outport
  theta : Outport  Port=2
  |U| : Outport  Port=3
  wires:
  Ualpha/1 -> Real-Imag to Complex/1
  Ubeta/1 -> Real-Imag to Complex/2
  Real-Imag to Complex/1 -> Complex to Magnitude-Angle/1
  Complex to Magnitude-Angle/2 -> Scope4/1, Sum/1
  Constant/1 -> Sum/2
  Sum/1 -> Scope2/1, MATLAB Function/1(u), MATLAB Function6/1(u), MATLAB Function5/1(u), MATLAB Function4/1(u), MATLAB Function3/1(u), MATLAB Function2/1(u), MATLAB Function1/1(u)
  MATLAB Function/1(y) -> Ceil/1
  Ceil/1 -> Multiport Switch1/1, Multiport Switch/1
  Multiport Switch/1 -> Scope1/1, theta/1
  MATLAB Function1/1(y) -> Multiport Switch/2
  MATLAB Function2/1(y) -> Multiport Switch/3
  MATLAB Function3/1(y) -> Multiport Switch/4
  MATLAB Function4/1(y) -> Multiport Switch/5
  MATLAB Function5/1(y) -> Multiport Switch/6
  MATLAB Function6/1(y) -> Multiport Switch/7
  Multiport Switch1/1 -> Scope/1, N/1
  Constant1/1 -> Multiport Switch1/5
  Constant2/1 -> Multiport Switch1/6
  Constant3/1 -> Multiport Switch1/7
  Constant4/1 -> Multiport Switch1/2
  Constant5/1 -> Multiport Switch1/3
  Constant6/1 -> Multiport Switch1/4
  Complex to Magnitude-Angle/1 -> |U|/1, Scope3/1
